simulink model "MX_Gateway"  (Simulink 9.0)
settings: Solver=FixedStepDiscrete  StartTime=0.0  StopTime=100  FixedStep=0.01  RelTol=1e-3
values from neighbouring files (symbols this model uses):
  ACTIVE = Bobcat.Parameter; CreateDefine( ACTIVE                         ,1                          ,'Define' ,'defines.h' )    [defines.m]
  ACTIVE_MODE_ONE = Bobcat.Parameter; CreateDefine( ACTIVE_MODE_ONE                ,19                         ,'Define' ,'defines.h' )    [defines.m]
  AUTHENTICATED = Bobcat.Parameter; CreateDefine( AUTHENTICATED                  ,1                          ,'Define' ,'defines.h' )    [defines.m]
  AUX_EMEA_CONTROLS = Bobcat.Parameter; CreateDefine( AUX_EMEA_CONTROLS  ,1   ,'Define' ,'defines.h' )    [enums.m]
  AUX_FLOW_HIGH = Bobcat.Parameter; CreateDefine( AUX_FLOW_HIGH           ,4  ,'Define' ,'defines.h' )    [enums.m]
  AUX_FLOW_INACTIVE = Bobcat.Parameter; CreateDefine( AUX_FLOW_INACTIVE       ,0  ,'Define' ,'defines.h' )    [enums.m]
  AUX_FLOW_LOW = Bobcat.Parameter; CreateDefine( AUX_FLOW_LOW            ,1  ,'Define' ,'defines.h' )    [enums.m]
  AUX_FLOW_MEDIUM = Bobcat.Parameter; CreateDefine( AUX_FLOW_MEDIUM         ,2  ,'Define' ,'defines.h' )    [enums.m]
  AUX_FLOW_STANDARD = Bobcat.Parameter; CreateDefine( AUX_FLOW_STANDARD       ,3  ,'Define' ,'defines.h' )    [enums.m]
  AUX_NON_FUNCTIONAL = Bobcat.Parameter; CreateDefine( AUX_NON_FUNCTIONAL ,255 ,'Define' ,'defines.h' )    [enums.m]
  BASE_ACTIVE = Bobcat.Parameter; CreateDefine( BASE_ACTIVE                    ,253                        ,'Define' ,'defines.h' )    [defines.m]
  BASE_DETENT = Bobcat.Parameter; CreateDefine( BASE_DETENT                    ,1                          ,'Define' ,'defines.h' )    [defines.m]
  BEEP_NONE = Bobcat.Parameter; CreateDefine( BEEP_NONE                      ,255                        ,'Define' ,'defines.h' )    [defines.m]
  CD_AIR_FILTER_DEBOUNCE_NORMAL = Bobcat.Parameter; CreateAliasCD( CD_AIR_FILTER_DEBOUNCE_NORMAL                        ,uint16(500)                ,'ConstantDataPointer' ,'CONSTANT_DATA' ,'AIR_FILTER_DEBOUNCE_NORMAL')    [cdata.m]    (also in hc12_cdata.m; m4_cdata.m)
  CD_AIR_FILTER_DEBOUNCE_NOT_CONNECTED = Bobcat.Parameter; CreateAliasCD( CD_AIR_FILTER_DEBOUNCE_NOT_CONNECTED                 ,uint16(3000)               ,'ConstantDataPointer' ,'CONSTANT_DATA' ,'AIR_FILTER_DEBOUNCE_NOT_CONNECTED')    [cdata.m]    (also in hc12_cdata.m; m4_cdata.m)
  CD_AIR_FILTER_DEBOUNCE_PLUGGED = Bobcat.Parameter; CreateAliasCD( CD_AIR_FILTER_DEBOUNCE_PLUGGED                       ,uint16(3000)               ,'ConstantDataPointer' ,'CONSTANT_DATA' ,'AIR_FILTER_DEBOUNCE_PLUGGED')    [cdata.m]    (also in hc12_cdata.m; m4_cdata.m)
  CD_AIR_FILTER_FUNCTION = Bobcat.Parameter; CreateAliasCD( CD_AIR_FILTER_FUNCTION                               ,uint8(255)                 ,'ConstantDataPointer' ,'CONSTANT_DATA' ,'AIR_FILTER_FUNCTION')    [cdata.m]    (also in hc12_cdata.m; m4_cdata.m)
  CD_AIR_FILTER_PLUGGED_WEIGHT = Bobcat.Parameter; CreateAliasCD( CD_AIR_FILTER_PLUGGED_WEIGHT                         ,uint8(2)                   ,'ConstantDataPointer' ,'CONSTANT_DATA' ,'AIR_FILTER_PLUGGED_WEIGHT')    [cdata.m]    (also in hc12_cdata.m; m4_cdata.m)
  CD_AIR_FILTER_UNPLUGGED_WEIGHT = Bobcat.Parameter; CreateAliasCD( CD_AIR_FILTER_UNPLUGGED_WEIGHT                       ,uint8(1)                   ,'ConstantDataPointer' ,'CONSTANT_DATA' ,'AIR_FILTER_UNPLUGGED_WEIGHT')    [cdata.m]    (also in hc12_cdata.m; m4_cdata.m)
  CD_AUTOIDLE_ARMBAR_DELAY = Bobcat.Parameter; CreateAliasCD( CD_AUTOIDLE_ARMBAR_DELAY                             ,uint8(2)                   ,'ConstantDataPointer' ,'CONSTANT_DATA' ,'AUTOIDLE_ARMBAR_DELAY')    [cdata.m]    (also in hc12_cdata.m; m4_cdata.m)
  CD_AUTOIDLE_FUNCTION = Bobcat.Parameter; CreateAliasCD( CD_AUTOIDLE_FUNCTION                                 ,uint8(255)                 ,'ConstantDataPointer' ,'CONSTANT_DATA' ,'AUTOIDLE_FUNCTION')    [cdata.m]    (also in hc12_cdata.m; m4_cdata.m)
  CD_AUTOIDLE_IDLE_DELAY = Bobcat.Parameter; CreateAliasCD( CD_AUTOIDLE_IDLE_DELAY                               ,uint8(40)                  ,'ConstantDataPointer' ,'CONSTANT_DATA' ,'AUTOIDLE_IDLE_DELAY')    [cdata.m]    (also in hc12_cdata.m; m4_cdata.m)
  CD_AUTOIDLE_LOW_IDLE_START = Bobcat.Parameter; CreateAliasCD( CD_AUTOIDLE_LOW_IDLE_START                           ,uint8(255)                 ,'ConstantDataPointer' ,'CONSTANT_DATA' ,'AUTOIDLE_LOW_IDLE_START')    [cdata.m]    (also in hc12_cdata.m; m4_cdata.m)
  CD_AUTOIDLE_PRESSURE_OFF = Bobcat.Parameter; CreateAliasCD( CD_AUTOIDLE_PRESSURE_OFF                             ,uint16(300)                ,'ConstantDataPointer' ,'CONSTANT_DATA' ,'AUTOIDLE_PRESSURE_OFF')    [cdata.m]    (also in hc12_cdata.m; m4_cdata.m)
  CD_AUTOIDLE_PRESSURE_ON = Bobcat.Parameter; CreateAliasCD( CD_AUTOIDLE_PRESSURE_ON                              ,uint16(270)                ,'ConstantDataPointer' ,'CONSTANT_DATA' ,'AUTOIDLE_PRESSURE_ON')    [cdata.m]    (also in hc12_cdata.m; m4_cdata.m)
  CD_AUTOSHIFT_FUNCTION = Bobcat.Parameter; CreateAliasCD( CD_AUTOSHIFT_FUNCTION                                ,uint8(255)                 ,'ConstantDataPointer' ,'CONSTANT_DATA' ,'AUTOSHIFT_FUNCTION')    [cdata.m]    (also in hc12_cdata.m; m4_cdata.m)
  CD_AUTOSHIFT_PHIGH1 = Bobcat.Parameter; CreateAliasCD( CD_AUTOSHIFT_PHIGH1                                  ,uint16(205)                ,'ConstantDataPointer' ,'CONSTANT_DATA' ,'AUTOSHIFT_PHIGH1')    [cdata.m]    (also in hc12_cdata.m; m4_cdata.m)
  CD_AUTOSHIFT_PHIGH2 = Bobcat.Parameter; CreateAliasCD( CD_AUTOSHIFT_PHIGH2                                  ,uint16(193)                ,'ConstantDataPointer' ,'CONSTANT_DATA' ,'AUTOSHIFT_PHIGH2')    [cdata.m]    (also in hc12_cdata.m; m4_cdata.m)
  CD_AUTOSHIFT_PHIGH_TEMP1 = Bobcat.Parameter; CreateAliasCD( CD_AUTOSHIFT_PHIGH_TEMP1                             ,int16(70)                  ,'ConstantDataPointer' ,'CONSTANT_DATA' ,'AUTOSHIFT_PHIGH_TEMP1')    [cdata.m]    (also in hc12_cdata.m; m4_cdata.m)
  CD_AUTOSHIFT_PHIGH_TEMP2 = Bobcat.Parameter; CreateAliasCD( CD_AUTOSHIFT_PHIGH_TEMP2                             ,int16(160)                 ,'ConstantDataPointer' ,'CONSTANT_DATA' ,'AUTOSHIFT_PHIGH_TEMP2')    [cdata.m]    (also in hc12_cdata.m; m4_cdata.m)
  CD_AUTOSHIFT_PLOW1 = Bobcat.Parameter; CreateAliasCD( CD_AUTOSHIFT_PLOW1                                   ,uint16(130)                ,'ConstantDataPointer' ,'CONSTANT_DATA' ,'AUTOSHIFT_PLOW1')    [cdata.m]    (also in hc12_cdata.m; m4_cdata.m)
  CD_AUTOSHIFT_PLOW2 = Bobcat.Parameter; CreateAliasCD( CD_AUTOSHIFT_PLOW2                                   ,uint16(100)                ,'ConstantDataPointer' ,'CONSTANT_DATA' ,'AUTOSHIFT_PLOW2')    [cdata.m]    (also in hc12_cdata.m; m4_cdata.m)
  CD_AUTOSHIFT_PLOW_TEMP1 = Bobcat.Parameter; CreateAliasCD( CD_AUTOSHIFT_PLOW_TEMP1                              ,int16(20)                  ,'ConstantDataPointer' ,'CONSTANT_DATA' ,'AUTOSHIFT_PLOW_TEMP1')    [cdata.m]    (also in hc12_cdata.m; m4_cdata.m)
  CD_AUTOSHIFT_PLOW_TEMP2 = Bobcat.Parameter; CreateAliasCD( CD_AUTOSHIFT_PLOW_TEMP2                              ,int16(80)                  ,'ConstantDataPointer' ,'CONSTANT_DATA' ,'AUTOSHIFT_PLOW_TEMP2')    [cdata.m]    (also in hc12_cdata.m; m4_cdata.m)
  CD_AUTOSHIFT_TRAVEL_DELAY = Bobcat.Parameter; CreateAliasCD( CD_AUTOSHIFT_TRAVEL_DELAY                            ,uint8(32)                  ,'ConstantDataPointer' ,'CONSTANT_DATA' ,'AUTOSHIFT_TRAVEL_DELAY')    [cdata.m]    (also in hc12_cdata.m; m4_cdata.m)
  CD_AUX2_CRACKPOINT_CURRENT = Bobcat.Parameter; CreateAliasCD( CD_AUX2_CRACKPOINT_CURRENT                           ,fi(0,0,16,12,'hex','0c28') ,'ConstantDataPointer' ,'CONSTANT_DATA' ,'AUX2_CRACKPOINT_CURRENT')    [cdata.m]    (also in hc12_cdata.m; m4_cdata.m)
  CD_AUX2_FLOW_LOW_FUNCTION = Bobcat.Parameter; CreateAliasCD( CD_AUX2_FLOW_LOW_FUNCTION                            ,uint8(255)                 ,'ConstantDataPointer' ,'CONSTANT_DATA' ,'AUX2_FLOW_LOW_FUNCTION')    [cdata.m]    (also in hc12_cdata.m; m4_cdata.m)
  CD_AUX2_FLOW_MED_FUNCTION = Bobcat.Parameter; CreateAliasCD( CD_AUX2_FLOW_MED_FUNCTION                            ,uint8(255)                 ,'ConstantDataPointer' ,'CONSTANT_DATA' ,'AUX2_FLOW_MED_FUNCTION')    [cdata.m]    (also in hc12_cdata.m; m4_cdata.m)
  CD_AUX2_HI_FLOW_PERCENT = Bobcat.Parameter; CreateAliasCD( CD_AUX2_HI_FLOW_PERCENT                              ,fi(0,0,16,16,'hex','ffff') ,'ConstantDataPointer' ,'CONSTANT_DATA' ,'AUX2_HI_FLOW_PERCENT')    [cdata.m]    (also in hc12_cdata.m; m4_cdata.m)
  CD_AUX2_LOW_FLOW_PERCENT = Bobcat.Parameter; CreateAliasCD( CD_AUX2_LOW_FLOW_PERCENT                             ,fi(0,0,16,16,'hex','7333') ,'ConstantDataPointer' ,'CONSTANT_DATA' ,'AUX2_LOW_FLOW_PERCENT')    [cdata.m]    (also in hc12_cdata.m; m4_cdata.m)
  CD_AUX2_MAX_FLOW_CURRENT = Bobcat.Parameter; CreateAliasCD( CD_AUX2_MAX_FLOW_CURRENT                             ,fi(0,0,16,12,'hex','14cc') ,'ConstantDataPointer' ,'CONSTANT_DATA' ,'AUX2_MAX_FLOW_CURRENT')    [cdata.m]    (also in hc12_cdata.m; m4_cdata.m)
  CD_AUX2_MED_FLOW_PERCENT = Bobcat.Parameter; CreateAliasCD( CD_AUX2_MED_FLOW_PERCENT                             ,fi(0,0,16,16,'hex','9999') ,'ConstantDataPointer' ,'CONSTANT_DATA' ,'AUX2_MED_FLOW_PERCENT')    [cdata.m]    (also in hc12_cdata.m; m4_cdata.m)
  CD_AUX_CRACKPOINT_CURRENT = Bobcat.Parameter; CreateAliasCD( CD_AUX_CRACKPOINT_CURRENT                            ,fi(0,0,16,12,'hex','0000') ,'ConstantDataPointer' ,'CONSTANT_DATA' ,'AUX_CRACKPOINT_CURRENT')    [cdata.m]    (also in hc12_cdata.m; m4_cdata.m)
  CD_AUX_DETENT_FLASH_TIME = Bobcat.Parameter; CreateAliasCD( CD_AUX_DETENT_FLASH_TIME                             ,uint16(72)                 ,'ConstantDataPointer' ,'CONSTANT_DATA' ,'AUX_DETENT_FLASH_TIME')    [cdata.m]    (also in hc12_cdata.m; m4_cdata.m)
  CD_AUX_DETENT_HOLD_TIME = Bobcat.Parameter; CreateAliasCD( CD_AUX_DETENT_HOLD_TIME                              ,uint16(100)                ,'ConstantDataPointer' ,'CONSTANT_DATA' ,'AUX_DETENT_HOLD_TIME')    [cdata.m]    (also in hc12_cdata.m; m4_cdata.m)
  CD_AUX_FULL_ON_AUX1 = Bobcat.Parameter; CreateAliasCD( CD_AUX_FULL_ON_AUX1                                  ,fi(0,0,16,12,'hex','10cd') ,'ConstantDataPointer' ,'CONSTANT_DATA' ,'AUX_FULL_ON_AUX1')    [cdata.m]    (also in hc12_cdata.m; m4_cdata.m)
  CD_AUX_FULL_ON_AUX2 = Bobcat.Parameter; CreateAliasCD( CD_AUX_FULL_ON_AUX2                                  ,fi(0,0,16,12,'hex','139d') ,'ConstantDataPointer' ,'CONSTANT_DATA' ,'AUX_FULL_ON_AUX2')    [cdata.m]    (also in hc12_cdata.m; m4_cdata.m)
  CD_AUX_FULL_ON_AUX3 = Bobcat.Parameter; CreateAliasCD( CD_AUX_FULL_ON_AUX3                                  ,fi(0,0,16,12,'hex','17d7') ,'ConstantDataPointer' ,'CONSTANT_DATA' ,'AUX_FULL_ON_AUX3')    [cdata.m]    (also in hc12_cdata.m; m4_cdata.m)
  CD_AUX_FUNCTION = Bobcat.Parameter; CreateAliasCD( CD_AUX_FUNCTION                                      ,uint8(0)                   ,'ConstantDataPointer' ,'CONSTANT_DATA' ,'AUX_FUNCTION')    [cdata.m]    (also in hc12_cdata.m; m4_cdata.m)
  CD_AUX_RATE_LIMIT_CRACKPOINT = Bobcat.Parameter; CreateAliasCD( CD_AUX_RATE_LIMIT_CRACKPOINT                         ,fi(0,0,16,12,'hex','0800') ,'ConstantDataPointer' ,'CONSTANT_DATA' ,'AUX_RATE_LIMIT_CRACKPOINT')    [cdata.m]    (also in hc12_cdata.m; m4_cdata.m)
  CD_AUX_RATE_LIMIT_CRACKPOINT_RATE = Bobcat.Parameter; CreateAliasCD( CD_AUX_RATE_LIMIT_CRACKPOINT_RATE                    ,fi(0,1,32,12,'int',102400) ,'ConstantDataPointer' ,'CONSTANT_DATA' ,'AUX_RATE_LIMIT_CRACKPOINT_RATE')    [cdata.m]    (also in hc12_cdata.m; m4_cdata.m)
  CD_AUX_RATE_LIMIT_FUNCTION = Bobcat.Parameter; CreateAliasCD( CD_AUX_RATE_LIMIT_FUNCTION                           ,uint8(255)                 ,'ConstantDataPointer' ,'CONSTANT_DATA' ,'AUX_RATE_LIMIT_FUNCTION')    [cdata.m]    (also in hc12_cdata.m; m4_cdata.m)
  CD_AUX_RATE_LIMIT_MAX_ENG_SPD = Bobcat.Parameter; CreateAliasCD( CD_AUX_RATE_LIMIT_MAX_ENG_SPD                        ,uint16(1600)               ,'ConstantDataPointer' ,'CONSTANT_DATA' ,'AUX_RATE_LIMIT_MAX_ENG_SPD')    [cdata.m]    (also in hc12_cdata.m; m4_cdata.m)
  CD_AUX_RATE_LIMIT_MIN_ENG_SPD = Bobcat.Parameter; CreateAliasCD( CD_AUX_RATE_LIMIT_MIN_ENG_SPD                        ,uint16(100)                ,'ConstantDataPointer' ,'CONSTANT_DATA' ,'AUX_RATE_LIMIT_MIN_ENG_SPD')    [cdata.m]    (also in hc12_cdata.m; m4_cdata.m)
  CD_AUX_RATE_LIMIT_NORMAL_RATE = Bobcat.Parameter; CreateAliasCD( CD_AUX_RATE_LIMIT_NORMAL_RATE                        ,fi(0,1,32,12,'int',2048)   ,'ConstantDataPointer' ,'CONSTANT_DATA' ,'AUX_RATE_LIMIT_NORMAL_RATE')    [cdata.m]    (also in hc12_cdata.m; m4_cdata.m)
  CD_AUXPOT_CAL_DEADBAND_HIGH = Bobcat.Parameter; CreateAliasCD( CD_AUXPOT_CAL_DEADBAND_HIGH                          ,uint16(574)                ,'ConstantDataPointer' ,'CONSTANT_DATA' ,'AUXPOT_CAL_DEADBAND_HIGH')    [cdata.m]    (also in hc12_cdata.m; m4_cdata.m)
  CD_AUXPOT_CAL_DEADBAND_LOW = Bobcat.Parameter; CreateAliasCD( CD_AUXPOT_CAL_DEADBAND_LOW                           ,uint16(424)                ,'ConstantDataPointer' ,'CONSTANT_DATA' ,'AUXPOT_CAL_DEADBAND_LOW')    [cdata.m]    (also in hc12_cdata.m; m4_cdata.m)
  CD_AUXPOT_CENTER_RATIO = Bobcat.Parameter; CreateAliasCD( CD_AUXPOT_CENTER_RATIO                               ,uint16(500)                ,'ConstantDataPointer' ,'CONSTANT_DATA' ,'AUXPOT_CENTER_RATIO')    [cdata.m]    (also in hc12_cdata.m; m4_cdata.m)
  CD_AUXPOT_DEADBAND = Bobcat.Parameter; CreateAliasCD( CD_AUXPOT_DEADBAND                                   ,uint8(38)                  ,'ConstantDataPointer' ,'CONSTANT_DATA' ,'AUXPOT_DEADBAND')    [cdata.m]    (also in hc12_cdata.m; m4_cdata.m)
  CD_AUXPOT_FUNCTION = Bobcat.Parameter; CreateAliasCD( CD_AUXPOT_FUNCTION                                   ,uint8(0)                   ,'ConstantDataPointer' ,'CONSTANT_DATA' ,'AUXPOT_FUNCTION')    [cdata.m]    (also in hc12_cdata.m; m4_cdata.m)
  CD_AUXPOT_RATIO_GAIN = Bobcat.Parameter; CreateAliasCD( CD_AUXPOT_RATIO_GAIN                                 ,uint16(1000)               ,'ConstantDataPointer' ,'CONSTANT_DATA' ,'AUXPOT_RATIO_GAIN')    [cdata.m]    (also in hc12_cdata.m; m4_cdata.m)
  CD_CAN_THROTTLE_FUNCTION = Bobcat.Parameter; CreateAliasCD( CD_CAN_THROTTLE_FUNCTION                             ,uint8(255)                 ,'ConstantDataPointer' ,'CONSTANT_DATA' ,'CAN_THROTTLE_FUNCTION')    [cdata.m]    (also in hc12_cdata.m; m4_cdata.m)
  CD_CAN_THROTTLE_REVERSE = Bobcat.Parameter; CreateAliasCD( CD_CAN_THROTTLE_REVERSE                              ,uint8(255)                 ,'ConstantDataPointer' ,'CONSTANT_DATA' ,'CAN_THROTTLE_REVERSE')    [cdata.m]    (also in hc12_cdata.m; m4_cdata.m)
  CD_CONSOLE_DEBOUNCE_ABSENT = Bobcat.Parameter; CreateAliasCD( CD_CONSOLE_DEBOUNCE_ABSENT                           ,uint16(12)                 ,'ConstantDataPointer' ,'CONSTANT_DATA' ,'CONSOLE_DEBOUNCE_ABSENT')    [cdata.m]    (also in hc12_cdata.m; m4_cdata.m)
  CD_CONSOLE_DEBOUNCE_OORH = Bobcat.Parameter; CreateAliasCD( CD_CONSOLE_DEBOUNCE_OORH                             ,uint16(12)                 ,'ConstantDataPointer' ,'CONSTANT_DATA' ,'CONSOLE_DEBOUNCE_OORH')    [cdata.m]    (also in hc12_cdata.m; m4_cdata.m)
  CD_CONSOLE_DEBOUNCE_OORL = Bobcat.Parameter; CreateAliasCD( CD_CONSOLE_DEBOUNCE_OORL                             ,uint16(12)                 ,'ConstantDataPointer' ,'CONSTANT_DATA' ,'CONSOLE_DEBOUNCE_OORL')    [cdata.m]    (also in hc12_cdata.m; m4_cdata.m)
  CD_CONSOLE_DEBOUNCE_PRESENT = Bobcat.Parameter; CreateAliasCD( CD_CONSOLE_DEBOUNCE_PRESENT                          ,uint16(12)                 ,'ConstantDataPointer' ,'CONSTANT_DATA' ,'CONSOLE_DEBOUNCE_PRESENT')    [cdata.m]    (also in hc12_cdata.m; m4_cdata.m)
  CD_CONSOLE_FUNCTION = Bobcat.Parameter; CreateAliasCD( CD_CONSOLE_FUNCTION                                  ,uint8(0)                   ,'ConstantDataPointer' ,'CONSTANT_DATA' ,'CONSOLE_FUNCTION')    [cdata.m]    (also in hc12_cdata.m; m4_cdata.m)
  CD_CONSOLE_OORH = Bobcat.Parameter; CreateAliasCD( CD_CONSOLE_OORH                                      ,uint16(740)                ,'ConstantDataPointer' ,'CONSTANT_DATA' ,'CONSOLE_OORH')    [cdata.m]    (also in hc12_cdata.m; m4_cdata.m)
  CD_CONSOLE_OORL = Bobcat.Parameter; CreateAliasCD( CD_CONSOLE_OORL                                      ,uint16(10)                 ,'ConstantDataPointer' ,'CONSTANT_DATA' ,'CONSOLE_OORL')    [cdata.m]    (also in hc12_cdata.m; m4_cdata.m)
  CD_CONSOLE_PRESENT_HIGH = Bobcat.Parameter; CreateAliasCD( CD_CONSOLE_PRESENT_HIGH                              ,uint16(515)                ,'ConstantDataPointer' ,'CONSTANT_DATA' ,'CONSOLE_PRESENT_HIGH')    [cdata.m]    (also in hc12_cdata.m; m4_cdata.m)
  CD_CONSOLE_PRESENT_LOW = Bobcat.Parameter; CreateAliasCD( CD_CONSOLE_PRESENT_LOW                               ,uint16(375)                ,'ConstantDataPointer' ,'CONSTANT_DATA' ,'CONSOLE_PRESENT_LOW')    [cdata.m]    (also in hc12_cdata.m; m4_cdata.m)
  CD_DELUXE_GEN5_5_FUNCTION = Bobcat.Parameter; CreateAliasCD( CD_DELUXE_GEN5_5_FUNCTION                            ,uint8(255)                 ,'ConstantDataPointer' ,'CONSTANT_DATA' ,'DELUXE_GEN5_5_FUNCTION')    [cdata.m]    (also in hc12_cdata.m; m4_cdata.m)
  CD_DELUXE_GEN5_FUNCTION = Bobcat.Parameter; CreateAliasCD( CD_DELUXE_GEN5_FUNCTION                              ,uint8(255)                 ,'ConstantDataPointer' ,'CONSTANT_DATA' ,'DELUXE_GEN5_FUNCTION')    [cdata.m]    (also in hc12_cdata.m; m4_cdata.m)
  CD_DERATE_ENGINE_SPEED_DEBOUNCE_HIGH = Bobcat.Parameter; CreateAliasCD( CD_DERATE_ENGINE_SPEED_DEBOUNCE_HIGH                 ,uint16(200)                ,'ConstantDataPointer' ,'CONSTANT_DATA' ,'DERATE_ENGINE_SPEED_DEBOUNCE_HIGH')    [cdata.m]    (also in hc12_cdata.m; m4_cdata.m)
  CD_DERATE_ENGINE_SPEED_DEBOUNCE_SHUTDOWN = Bobcat.Parameter; CreateAliasCD( CD_DERATE_ENGINE_SPEED_DEBOUNCE_SHUTDOWN             ,uint16(1000)               ,'ConstantDataPointer' ,'CONSTANT_DATA' ,'DERATE_ENGINE_SPEED_DEBOUNCE_SHUTDOWN')    [cdata.m]    (also in hc12_cdata.m; m4_cdata.m)
  CD_DERATE_ENGINE_SPEED_HIGH_OFFSET = Bobcat.Parameter; CreateAliasCD( CD_DERATE_ENGINE_SPEED_HIGH_OFFSET                   ,uint16(200)                ,'ConstantDataPointer' ,'CONSTANT_DATA' ,'DERATE_ENGINE_SPEED_HIGH_OFFSET')    [cdata.m]    (also in hc12_cdata.m; m4_cdata.m)
  CD_DERATE_METHOD = Bobcat.Parameter; CreateAliasCD( CD_DERATE_METHOD                                     ,uint8(0)                   ,'ConstantDataPointer' ,'CONSTANT_DATA' ,'DERATE_METHOD')    [cdata.m]    (also in hc12_cdata.m; m4_cdata.m)
  CD_DUMP_VALVE_FUNCTION = Bobcat.Parameter; CreateAliasCD( CD_DUMP_VALVE_FUNCTION                               ,uint8(255)                 ,'ConstantDataPointer' ,'CONSTANT_DATA' ,'DUMP_VALVE_FUNCTION')    [cdata.m]    (also in hc12_cdata.m; m4_cdata.m)
  CD_DUMP_VALVE_ON_CURRENT = Bobcat.Parameter; CreateAliasCD( CD_DUMP_VALVE_ON_CURRENT                             ,fi(0,0,16,12,'hex','ffff') ,'ConstantDataPointer' ,'CONSTANT_DATA' ,'DUMP_VALVE_ON_CURRENT')    [cdata.m]    (also in hc12_cdata.m; m4_cdata.m)
  CD_ECO_MODE_THROTTLE_REDUCTION = Bobcat.Parameter; CreateAliasCD( CD_ECO_MODE_THROTTLE_REDUCTION                       ,uint16(850)                ,'ConstantDataPointer' ,'CONSTANT_DATA' ,'ECO_MODE_THROTTLE_REDUCTION')    [cdata.m]    (also in hc12_cdata.m; m4_cdata.m)
  CD_ECT_FILTER_GAIN = Bobcat.Parameter; CreateAliasCD( CD_ECT_FILTER_GAIN                                   ,uint8(6)                   ,'ConstantDataPointer' ,'CONSTANT_DATA' ,'ECT_FILTER_GAIN')    [cdata.m]    (also in hc12_cdata.m; m4_cdata.m)
  CD_ECU_FUNCTION = Bobcat.Parameter; CreateAliasCD( CD_ECU_FUNCTION                                      ,uint8(255)                 ,'ConstantDataPointer' ,'CONSTANT_DATA' ,'ECU_FUNCTION')    [cdata.m]    (also in hc12_cdata.m; m4_cdata.m)
  CD_ECU_HOLD_DEBOUNCE_ERROR_ON = Bobcat.Parameter; CreateAliasCD( CD_ECU_HOLD_DEBOUNCE_ERROR_ON                        ,uint16(20)                 ,'ConstantDataPointer' ,'CONSTANT_DATA' ,'ECU_HOLD_DEBOUNCE_ERROR_ON')    [cdata.m]    (also in hc12_cdata.m; m4_cdata.m)
  CD_EMEA_CONTROLS_HOLD_TIME = Bobcat.Parameter; CreateAliasCD( CD_EMEA_CONTROLS_HOLD_TIME                           ,uint16(30)                 ,'ConstantDataPointer' ,'CONSTANT_DATA' ,'EMEA_CONTROLS_HOLD_TIME')    [cdata.m]    (also in hc12_cdata.m; m4_cdata.m)
  CD_EMEA_CONTROLS_OPTION_A = Bobcat.Parameter; CreateAliasCD( CD_EMEA_CONTROLS_OPTION_A                            ,uint8(255)                 ,'ConstantDataPointer' ,'CONSTANT_DATA' ,'EMEA_CONTROLS_OPTION_A')    [cdata.m]    (also in hc12_cdata.m; m4_cdata.m)
  CD_EMEA_CONTROLS_OPTION_C = Bobcat.Parameter; CreateAliasCD( CD_EMEA_CONTROLS_OPTION_C                            ,uint8(255)                 ,'ConstantDataPointer' ,'CONSTANT_DATA' ,'EMEA_CONTROLS_OPTION_C')    [cdata.m]    (also in hc12_cdata.m; m4_cdata.m)
  CD_EMEA_CONTROLS_REVERSE = Bobcat.Parameter; CreateAliasCD( CD_EMEA_CONTROLS_REVERSE                             ,uint8(255)                 ,'ConstantDataPointer' ,'CONSTANT_DATA' ,'EMEA_CONTROLS_REVERSE')    [cdata.m]    (also in hc12_cdata.m; m4_cdata.m)
  CD_EMEA_ELECTRIC_VERSION = Bobcat.Parameter; CreateAliasCD( CD_EMEA_ELECTRIC_VERSION                             ,uint8(0)                   ,'ConstantDataPointer' ,'CONSTANT_DATA' ,'EMEA_ELECTRIC_VERSION')    [cdata.m]    (also in hc12_cdata.m; m4_cdata.m)
  CD_EMEA_POT_CAL_DEADBAND_HIGH = Bobcat.Parameter; CreateAliasCD( CD_EMEA_POT_CAL_DEADBAND_HIGH                        ,uint16(574)                ,'ConstantDataPointer' ,'CONSTANT_DATA' ,'EMEA_POT_CAL_DEADBAND_HIGH')    [cdata.m]    (also in hc12_cdata.m; m4_cdata.m)
  CD_EMEA_POT_CAL_DEADBAND_LOW = Bobcat.Parameter; CreateAliasCD( CD_EMEA_POT_CAL_DEADBAND_LOW                         ,uint16(424)                ,'ConstantDataPointer' ,'CONSTANT_DATA' ,'EMEA_POT_CAL_DEADBAND_LOW')    [cdata.m]    (also in hc12_cdata.m; m4_cdata.m)
  CD_ENGINE_RPM_FACTOR = Bobcat.Parameter; CreateAliasCD( CD_ENGINE_RPM_FACTOR                                 ,uint8(35)                  ,'ConstantDataPointer' ,'CONSTANT_DATA' ,'ENGINE_RPM_FACTOR')    [cdata.m]    (also in hc12_cdata.m; m4_cdata.m)
  CD_ENGINE_SPEED_FILTER_FAST_VALUE = Bobcat.Parameter; CreateAliasCD( CD_ENGINE_SPEED_FILTER_FAST_VALUE                    ,uint8(1)                   ,'ConstantDataPointer' ,'CONSTANT_DATA' ,'ENGINE_SPEED_FILTER_FAST_VALUE')    [cdata.m]    (also in hc12_cdata.m; m4_cdata.m)
  CD_ENGINE_SPEED_FILTER_SLOW_VALUE = Bobcat.Parameter; CreateAliasCD( CD_ENGINE_SPEED_FILTER_SLOW_VALUE                    ,uint8(4)                   ,'ConstantDataPointer' ,'CONSTANT_DATA' ,'ENGINE_SPEED_FILTER_SLOW_VALUE')    [cdata.m]    (also in hc12_cdata.m; m4_cdata.m)
  CD_ENGINE_SPEED_RUNNING = Bobcat.Parameter; CreateAliasCD( CD_ENGINE_SPEED_RUNNING                              ,uint16(800)                ,'ConstantDataPointer' ,'CONSTANT_DATA' ,'ENGINE_SPEED_RUNNING')    [cdata.m]    (also in hc12_cdata.m; m4_cdata.m)
  CD_ENGINE_START_DELAY_DEBOUNCE_RPM_NO_SIGNAL = Bobcat.Parameter; CreateAliasCD( CD_ENGINE_START_DELAY_DEBOUNCE_RPM_NO_SIGNAL         ,uint16(0)                  ,'ConstantDataPointer' ,'CONSTANT_DATA' ,'ENGINE_START_DELAY_DEBOUNCE_RPM_NO_SIGNAL')    [cdata.m]    (also in hc12_cdata.m; m4_cdata.m)
  CD_ENGINE_START_DELAY_OFF_TIME = Bobcat.Parameter; CreateAliasCD( CD_ENGINE_START_DELAY_OFF_TIME                       ,uint16(0)                  ,'ConstantDataPointer' ,'CONSTANT_DATA' ,'ENGINE_START_DELAY_OFF_TIME')    [cdata.m]    (also in hc12_cdata.m; m4_cdata.m)
  CD_ENGINE_START_DELAY_RPM_NO_SIGNAL = Bobcat.Parameter; CreateAliasCD( CD_ENGINE_START_DELAY_RPM_NO_SIGNAL                  ,uint16(0)                  ,'ConstantDataPointer' ,'CONSTANT_DATA' ,'ENGINE_START_DELAY_RPM_NO_SIGNAL')    [cdata.m]    (also in hc12_cdata.m; m4_cdata.m)
  CD_EOP_DEBOUNCE_EXTREMELY_LOW = Bobcat.Parameter; CreateAliasCD( CD_EOP_DEBOUNCE_EXTREMELY_LOW                        ,uint16(50)                 ,'ConstantDataPointer' ,'CONSTANT_DATA' ,'EOP_DEBOUNCE_EXTREMELY_LOW')    [cdata.m]    (also in hc12_cdata.m; m4_cdata.m)
  CD_EOP_DEBOUNCE_NORMAL = Bobcat.Parameter; CreateAliasCD( CD_EOP_DEBOUNCE_NORMAL                               ,uint16(50)                 ,'ConstantDataPointer' ,'CONSTANT_DATA' ,'EOP_DEBOUNCE_NORMAL')    [cdata.m]    (also in hc12_cdata.m; m4_cdata.m)
  CD_EOP_DEBOUNCE_SHUTDOWN = Bobcat.Parameter; CreateAliasCD( CD_EOP_DEBOUNCE_SHUTDOWN                             ,uint16(100)                ,'ConstantDataPointer' ,'CONSTANT_DATA' ,'EOP_DEBOUNCE_SHUTDOWN')    [cdata.m]    (also in hc12_cdata.m; m4_cdata.m)
  CD_EOP_FILTER_VALUE = Bobcat.Parameter; CreateAliasCD( CD_EOP_FILTER_VALUE                                  ,uint8(3)                   ,'ConstantDataPointer' ,'CONSTANT_DATA' ,'EOP_FILTER_VALUE')    [cdata.m]    (also in hc12_cdata.m; m4_cdata.m)
  CD_EOP_FUNCTION = Bobcat.Parameter; CreateAliasCD( CD_EOP_FUNCTION                                      ,uint8(1)                   ,'ConstantDataPointer' ,'CONSTANT_DATA' ,'EOP_FUNCTION')    [cdata.m]    (also in hc12_cdata.m; m4_cdata.m)
  CD_EOP_SWITCH_GAUGE_VALUE = Bobcat.Parameter; CreateAliasCD( CD_EOP_SWITCH_GAUGE_VALUE                            ,uint16(125)                ,'ConstantDataPointer' ,'CONSTANT_DATA' ,'EOP_SWITCH_GAUGE_VALUE')    [cdata.m]    (also in hc12_cdata.m; m4_cdata.m)
  CD_EOP_SWITCH_LOW = Bobcat.Parameter; CreateAliasCD( CD_EOP_SWITCH_LOW                                    ,uint16(50)                 ,'ConstantDataPointer' ,'CONSTANT_DATA' ,'EOP_SWITCH_LOW')    [cdata.m]    (also in hc12_cdata.m; m4_cdata.m)
  CD_EOP_SWITCH_PRESSURE_VALUE = Bobcat.Parameter; CreateAliasCD( CD_EOP_SWITCH_PRESSURE_VALUE                         ,uint16(40)                 ,'ConstantDataPointer' ,'CONSTANT_DATA' ,'EOP_SWITCH_PRESSURE_VALUE')    [cdata.m]    (also in hc12_cdata.m; m4_cdata.m)
  CD_EOT_DEBOUNCE_EXTREMELY_LOW = Bobcat.Parameter; CreateAliasCD( CD_EOT_DEBOUNCE_EXTREMELY_LOW                        ,uint16(2)                  ,'ConstantDataPointer' ,'CONSTANT_DATA' ,'EOT_DEBOUNCE_EXTREMELY_LOW')    [cdata.m]    (also in hc12_cdata.m; m4_cdata.m)
  CD_EOT_DEBOUNCE_LOW = Bobcat.Parameter; CreateAliasCD( CD_EOT_DEBOUNCE_LOW                                  ,uint16(2)                  ,'ConstantDataPointer' ,'CONSTANT_DATA' ,'EOT_DEBOUNCE_LOW')    [cdata.m]    (also in hc12_cdata.m; m4_cdata.m)
  CD_EOT_DEBOUNCE_NORMAL = Bobcat.Parameter; CreateAliasCD( CD_EOT_DEBOUNCE_NORMAL                               ,uint16(2)                  ,'ConstantDataPointer' ,'CONSTANT_DATA' ,'EOT_DEBOUNCE_NORMAL')    [cdata.m]    (also in hc12_cdata.m; m4_cdata.m)
  CD_EOT_EXTREMELY_LOW = Bobcat.Parameter; CreateAliasCD( CD_EOT_EXTREMELY_LOW                                 ,uint16(81)                 ,'ConstantDataPointer' ,'CONSTANT_DATA' ,'EOT_EXTREMELY_LOW')    [cdata.m]    (also in hc12_cdata.m; m4_cdata.m)
  CD_EOT_FUNCTION = Bobcat.Parameter; CreateAliasCD( CD_EOT_FUNCTION                                      ,uint8(255)                 ,'ConstantDataPointer' ,'CONSTANT_DATA' ,'EOT_FUNCTION')    [cdata.m]    (also in hc12_cdata.m; m4_cdata.m)
  CD_EOT_LOW = Bobcat.Parameter; CreateAliasCD( CD_EOT_LOW                                           ,uint16(90)                 ,'ConstantDataPointer' ,'CONSTANT_DATA' ,'EOT_LOW')    [cdata.m]    (also in hc12_cdata.m; m4_cdata.m)
  CD_EOT_TEMPERATURE_HYSTERESIS = Bobcat.Parameter; CreateAliasCD( CD_EOT_TEMPERATURE_HYSTERESIS                        ,uint16(2)                  ,'ConstantDataPointer' ,'CONSTANT_DATA' ,'EOT_TEMPERATURE_HYSTERESIS')    [cdata.m]    (also in hc12_cdata.m; m4_cdata.m)
  CD_FAST_TRAVEL_FUNCTION = Bobcat.Parameter; CreateAliasCD( CD_FAST_TRAVEL_FUNCTION                              ,uint8(255)                 ,'ConstantDataPointer' ,'CONSTANT_DATA' ,'FAST_TRAVEL_FUNCTION')    [cdata.m]    (also in hc12_cdata.m; m4_cdata.m)
  CD_FAST_TRAVEL_THROTTLE_DELAY = Bobcat.Parameter; CreateAliasCD( CD_FAST_TRAVEL_THROTTLE_DELAY                        ,uint16(10)                 ,'ConstantDataPointer' ,'CONSTANT_DATA' ,'FAST_TRAVEL_THROTTLE_DELAY')    [cdata.m]    (also in hc12_cdata.m; m4_cdata.m)
  CD_FAST_TRAVEL_THROTTLE_LIMIT = Bobcat.Parameter; CreateAliasCD( CD_FAST_TRAVEL_THROTTLE_LIMIT                        ,uint16(1000)               ,'ConstantDataPointer' ,'CONSTANT_DATA' ,'FAST_TRAVEL_THROTTLE_LIMIT')    [cdata.m]    (also in hc12_cdata.m; m4_cdata.m)
  CD_FLYWHEEL_TEETH = Bobcat.Parameter; CreateAliasCD( CD_FLYWHEEL_TEETH                                    ,uint8(83)                  ,'ConstantDataPointer' ,'CONSTANT_DATA' ,'FLYWHEEL_TEETH')    [cdata.m]    (also in hc12_cdata.m; m4_cdata.m)
  CD_FUEL_LEVEL_FILTER_FAST_VALUE = Bobcat.Parameter; CreateAliasCD( CD_FUEL_LEVEL_FILTER_FAST_VALUE                      ,uint8(5)                   ,'ConstantDataPointer' ,'CONSTANT_DATA' ,'FUEL_LEVEL_FILTER_FAST_VALUE')    [cdata.m]    (also in hc12_cdata.m; m4_cdata.m)
  CD_FUEL_LEVEL_FILTER_SLOW_VALUE = Bobcat.Parameter; CreateAliasCD( CD_FUEL_LEVEL_FILTER_SLOW_VALUE                      ,uint8(10)                  ,'ConstantDataPointer' ,'CONSTANT_DATA' ,'FUEL_LEVEL_FILTER_SLOW_VALUE')    [cdata.m]    (also in hc12_cdata.m; m4_cdata.m)
  CD_FUEL_PRIMARY_FEEDBACK_FUNCTION = Bobcat.Parameter; CreateAliasCD( CD_FUEL_PRIMARY_FEEDBACK_FUNCTION                    ,uint8(0)                   ,'ConstantDataPointer' ,'CONSTANT_DATA' ,'FUEL_PRIMARY_FEEDBACK_FUNCTION')    [cdata.m]    (also in hc12_cdata.m; m4_cdata.m)
  ... (+246 more)
undefined symbols (not defined in the file or in its folder): sigbuilder_block
undefined symbols (not defined in the file): CD_FUEL_RESOLUTION_TIMER
libraries: simulink x186, MX_Library x107
(part 39 of 48)

== stateflow chart MX_Gtwy_Control/Inputs/AirFilter/Filter ==
  state PERSISTANCE_COUNTER [parallel]
    settings: executionOrder=1
    state COUNTER
      en:count_AFPlugged = 0;
      en:state_Engine_old = state_Engine;
      state OFF
        OFF -> (junction 4037) [order 1]
        (junction 4037) -> (junction 4038) [order 1] : [(count_AFPlugged >0)]
        (junction 4037) -> (junction 4039) [order 2]
        (junction 4038) -> (junction 4040) : {
            | count_AFPlugged = count_AFPlugged - CD_AIR_FILTER_UNPLUGGED_WEIGHT;
            | }
        (junction 4040) -> (junction 4039)
      state ON
        ON -> (junction 4046) [order 1]
        (junction 4046) -> (junction 4047) [order 1] : [(count_AFPlugged < 65535)]
        (junction 4046) -> (junction 4048) [order 2]
        (junction 4047) -> (junction 4049) : {
            | count_AFPlugged = count_AFPlugged + CD_AIR_FILTER_PLUGGED_WEIGHT;
            | }
        (junction 4049) -> (junction 4048)
      (default) -> OFF
      OFF -> ON [order 1] : [(din_AirFilter > 0)]
      ON -> OFF [order 1] : [(din_AirFilter == 0)]
    (default) -> COUNTER
    COUNTER -> COUNTER : [(state_Engine_old != state_Engine)]
  state FAULT_DETECTION [parallel]
    settings: executionOrder=2
    state NON_FUNCTIONAL
      en:state_AirFilter = NORMAL;
    state COUNTER_BASED
      state NOT_CONNECTED
        en:state_AirFilter = NOT_CONNECTED;
      state NORMAL
        en:state_AirFilter = NORMAL;
      state PLUGGED
        en:state_AirFilter = PLUGGED;
      (default) -> FAULT_DETECTION.COUNTER_BASED.NORMAL
      FAULT_DETECTION.COUNTER_BASED.NORMAL -> FAULT_DETECTION.COUNTER_BASED.NOT_CONNECTED [order 2] : [((state_Engine == ENGINE_NOT_RUNNING) || (state_AirFilter == NOT_CONNECTED))...
          | && (count_AFPlugged >= CD_AIR_FILTER_DEBOUNCE_NOT_CONNECTED)]
      FAULT_DETECTION.COUNTER_BASED.NOT_CONNECTED -> FAULT_DETECTION.COUNTER_BASED.NORMAL : [(count_AFPlugged < CD_AIR_FILTER_DEBOUNCE_NOT_CONNECTED)]
      FAULT_DETECTION.COUNTER_BASED.NORMAL -> FAULT_DETECTION.COUNTER_BASED.PLUGGED [order 1] : [(state_Engine == ENGINE_RUNNING)...
          | && (count_AFPlugged >= CD_AIR_FILTER_DEBOUNCE_PLUGGED)]
      FAULT_DETECTION.COUNTER_BASED.PLUGGED -> FAULT_DETECTION.COUNTER_BASED.NORMAL : [(count_AFPlugged < CD_AIR_FILTER_DEBOUNCE_PLUGGED)...
          | && (state_AirFilter == NORMAL)]
    state TIME_BASED
      state NOT_CONNECTED
        state NOT_CONNECTED
          en:state_AirFilter = NOT_CONNECTED;
        state DEBOUNCE
          en:debounceCount = 0;
          du:debounceCount++;
        (default) -> FAULT_DETECTION.TIME_BASED.NOT_CONNECTED.DEBOUNCE
        FAULT_DETECTION.TIME_BASED.NOT_CONNECTED.DEBOUNCE -> FAULT_DETECTION.TIME_BASED.NOT_CONNECTED.NOT_CONNECTED : [(debounceCount >= CD_AIR_FILTER_DEBOUNCE_NOT_CONNECTED)...
            | || (state_AirFilter == NOT_CONNECTED)]
      state NORMAL
        state DEBOUNCE
          en:debounceCount = 0;
          du:debounceCount++;
        state NORMAL
          en:state_AirFilter = NORMAL;
        (default) -> FAULT_DETECTION.TIME_BASED.NORMAL.DEBOUNCE
        FAULT_DETECTION.TIME_BASED.NORMAL.DEBOUNCE -> FAULT_DETECTION.TIME_BASED.NORMAL.NORMAL : [(debounceCount >= CD_AIR_FILTER_DEBOUNCE_NORMAL)...
            | || (state_AirFilter == NORMAL)]
      state PLUGGED
        state DEBOUNCE
          en:debounceCount = 0;
          du:debounceCount++;
        state PLUGGED
          en:state_AirFilter = PLUGGED;
        (default) -> FAULT_DETECTION.TIME_BASED.PLUGGED.DEBOUNCE
        FAULT_DETECTION.TIME_BASED.PLUGGED.DEBOUNCE -> FAULT_DETECTION.TIME_BASED.PLUGGED.PLUGGED : [(debounceCount >= CD_AIR_FILTER_DEBOUNCE_PLUGGED)]
      (default) -> FAULT_DETECTION.TIME_BASED.NORMAL
      FAULT_DETECTION.TIME_BASED.NORMAL -> FAULT_DETECTION.TIME_BASED.NOT_CONNECTED [order 2] : [(din_AirFilter == ACTIVE)...
          | && ((state_Engine == ENGINE_NOT_RUNNING) || (state_AirFilter == NOT_CONNECTED))]
      FAULT_DETECTION.TIME_BASED.NOT_CONNECTED -> FAULT_DETECTION.TIME_BASED.NORMAL : [(din_AirFilter != ACTIVE)]
      FAULT_DETECTION.TIME_BASED.NORMAL -> FAULT_DETECTION.TIME_BASED.PLUGGED [order 1] : [(din_AirFilter == ACTIVE)...
          | && (state_Engine == ENGINE_RUNNING)...
          | && (state_AirFilter != NOT_CONNECTED)]
      FAULT_DETECTION.TIME_BASED.PLUGGED -> FAULT_DETECTION.TIME_BASED.NORMAL : [(din_AirFilter != ACTIVE)...
          | && (state_AirFilter == NORMAL)]
    (default) -> NON_FUNCTIONAL
    NON_FUNCTIONAL -> COUNTER_BASED [order 1] : [(CD_AIR_FILTER_FUNCTION == 1)]
    NON_FUNCTIONAL -> TIME_BASED [order 2] : [(CD_AIR_FILTER_FUNCTION != NON_FUNCTIONAL)]
  data: din_AirFilter input uint8; state_Engine input uint8; state_AirFilter output uint8; CD_AIR_FILTER_DEBOUNCE_NORMAL parameter uint16; CD_AIR_FILTER_DEBOUNCE_NOT_CONNECTED parameter uint16; CD_AIR_FILTER_DEBOUNCE_PLUGGED parameter uint16; CD_AIR_FILTER_FUNCTION parameter uint8; debounceCount local uint16 =0; ACTIVE parameter uint8; ENGINE_NOT_RUNNING parameter uint8; PLUGGED parameter uint8; NON_FUNCTIONAL parameter uint8; ENGINE_RUNNING parameter uint8; NORMAL parameter uint8; NOT_CONNECTED parameter uint8; count_AFPlugged output uint16; state_Engine_old local uint8; CD_AIR_FILTER_PLUGGED_WEIGHT parameter uint8; CD_AIR_FILTER_UNPLUGGED_WEIGHT parameter uint8

== stateflow chart MX_Gtwy_Control/Inputs/Aux_Pot/Aux_Pot/Aux_Pot ==
  state Aux_Pot_Neutral_Check [parallel]
    settings: executionOrder=1
    state Initialize
      en:
      state_AuxPotCal = NORMAL;
      offset_AuxPotCal = 0;
    state WaitForPress
    state Wait_For_New_Cal
    note: if we are already NOT_IN_NEUTRAL
        | be sure to not beep again
    note: set the triple beep here instead of in the fault code
        | to be sure other aux beeps don't override the fault code beep
    (default) -> Initialize
    Initialize -> WaitForPress
    WaitForPress -> (junction 4113) [order 1] : [(aux_Flow == AUX_FLOW_LOW || aux_Flow == AUX_FLOW_MEDIUM || aux_Flow == AUX_FLOW_STANDARD || aux_Flow == AUX_FLOW_HIGH)...
        | && state_Console == ACTIVE...
        | && state_Engine != ENGINE_CRANKING...
        | && state_AuxPot != OORL...
        | && state_AuxPot != OORH...
        | && state_SensorSupply == NORMAL]
    (junction 4113) -> (junction 4114)
    (junction 4114) -> (junction 4115) [order 1] : [rm_AuxPot >= CD_AUXPOT_CAL_DEADBAND_LOW...
        |  && rm_AuxPot <= CD_AUXPOT_CAL_DEADBAND_HIGH]
    (junction 4118) -> (junction 4113)
    (junction 4114) -> (junction 4116) [order 2]
    (junction 4115) -> (junction 4119) : {
        | state_AuxPotCal = NORMAL;
        | offset_AuxPotCal = int16(CD_AUXPOT_CENTER_RATIO) - rm_AuxPot;
        | }
    (junction 4116) -> (junction 4117) [order 1] : [state_AuxPot != NOT_IN_NEUTRAL]
    (junction 4116) -> (junction 4120) [order 2] : {
        | state_Buzzer = BEEP_NONE;
        | }
    (junction 4117) -> (junction 4121) : {
        | state_AuxPotCal = NOT_IN_NEUTRAL;
        | offset_AuxPotCal = 0;
        | state_Buzzer = TRIPLE_SHORT_BEEP;
        | }
    WaitForPress -> (junction 4118) [order 2] : [(aux_DebounceButton || aux_DetentHold)...
        | && (state_AuxEnable == INACTIVE || aux_Flow == AUX_FLOW_INACTIVE)...
        | && state_AuxPot != OORL...
        | && state_AuxPot != OORH...
        | && state_SensorSupply == NORMAL]
    (junction 4122) -> (junction 4118)
    (junction 4119) -> Wait_For_New_Cal
    (junction 4120) -> (junction 4121)
    (junction 4121) -> Wait_For_New_Cal
    Wait_For_New_Cal -> (junction 4122) [order 2] : [(aux_DebounceButton || aux_DetentHold)...
        | && (state_AuxEnable == INACTIVE || aux_Flow == AUX_FLOW_INACTIVE)...
        | && state_AuxPot != OORL...
        | && state_AuxPot != OORH...
        | && state_SensorSupply == NORMAL]
    Wait_For_New_Cal -> WaitForPress [order 1] : [state_Console != ACTIVE...
        | || state_Engine == ENGINE_CRANKING...
        | || state_AuxPot == OORL ...
        | || state_AuxPot ==OORH ...
        | || state_SensorSupply != NORMAL]
  state Aux_Pot_State [parallel]
    settings: executionOrder=2
    state Supply_OK
      state NORMAL
        en:
        position_AuxPotIn = rm_AuxPot + offset_AuxPotCal;
        position_AuxPotOut = position_AuxPotIn;
        du:
        position_AuxPotIn = rm_AuxPot + offset_AuxPotCal;
        position_AuxPotOut = position_AuxPotIn;
        (history)
        state Neutral
          en:
          state_AuxPot = NEUTRAL;
        state Rod_Active
          en:
          state_AuxPot =...
          ROD_ACTIVE;
        state Base_Active
          en:
          state_AuxPot =...
          BASE_ACTIVE;
        (default) -> Neutral
        Neutral -> Base_Active [order 2] : [position_AuxPotIn < base_Threshold]
        Neutral -> Rod_Active [order 1] : [position_AuxPotIn > rod_Threshold]
        Rod_Active -> Neutral [order 2] : [position_AuxPotIn <= rod_Threshold]
        Base_Active -> Neutral [order 2] : [position_AuxPotIn >= base_Threshold]
        Rod_Active -> Base_Active [order 1] : [position_AuxPotIn <= rod_Threshold...
            |  && position_AuxPotIn < base_Threshold]
        Base_Active -> Rod_Active [order 1] : [position_AuxPotIn >= rod_Threshold...
            |  && position_AuxPotIn > base_Threshold]
      state OORH
        en:
        state_AuxPot = OORH;
      state debounce_OORH
        en:
        position_AuxPotOut =...
        CD_AUXPOT_CENTER_RATIO;
      state OORL
        en:
        state_AuxPot = OORL;
      state debounce_OORL
        en:
        position_AuxPotOut =...
        CD_AUXPOT_CENTER_RATIO;
      state NOT_IN_NEUTRAL
        en:
        state_AuxPot = NOT_IN_NEUTRAL;
        position_AuxPotOut =...
        CD_AUXPOT_CENTER_RATIO;
      (default) -> NORMAL
      NORMAL -> debounce_OORH [order 1] : [rm_AuxPot > CD_AUXPOT_OORH]
      debounce_OORH -> OORH [order 1] : [after(CD_AUXPOT_OOR_DB,tick)]
      debounce_OORH -> NORMAL [order 2] : [rm_AuxPot <= CD_AUXPOT_OORH]
      OORH -> NORMAL : [rm_AuxPot <= CD_AUXPOT_OORH]
      NORMAL -> debounce_OORL [order 2] : [rm_AuxPot < CD_AUXPOT_OORL]
      debounce_OORL -> OORL [order 1] : [after(CD_AUXPOT_OOR_DB,tick)]
      debounce_OORL -> NORMAL [order 2] : [rm_AuxPot >= CD_AUXPOT_OORL]
      OORL -> NORMAL : [rm_AuxPot >= CD_AUXPOT_OORL]
      NORMAL -> NOT_IN_NEUTRAL [order 3] : [state_AuxPotCal == NOT_IN_NEUTRAL]
      NOT_IN_NEUTRAL -> NORMAL : [state_AuxPotCal != NOT_IN_NEUTRAL]
    state Supply_Fault
      en:
      state_AuxPot = NORMAL;
      position_AuxPotOut =...
      CD_AUXPOT_CENTER_RATIO;
    (default) -> Supply_OK : {
        | base_Threshold = CD_AUXPOT_CENTER_RATIO - CD_AUXPOT_DEADBAND;
        | rod_Threshold = CD_AUXPOT_CENTER_RATIO + CD_AUXPOT_DEADBAND;
        | }
    Supply_OK -> Supply_Fault : [state_SensorSupply != NORMAL]
    Supply_Fault -> Supply_OK : [state_SensorSupply == NORMAL]
  data: aux_DebounceButton input uint8; aux_Flow input uint8; state_AuxEnable input uint8; aux_DetentHold input uint8; state_AuxPot output uint8; position_AuxPotOut output int16; position_AuxPotIn local int16 size=1; offset_AuxPotCal local int16; state_AuxPotCal local uint8; CD_AUXPOT_CENTER_RATIO parameter uint16; CD_AUXPOT_CAL_DEADBAND_HIGH parameter uint16; CD_AUXPOT_CAL_DEADBAND_LOW parameter uint16; CD_AUXPOT_OORH parameter uint16; CD_AUXPOT_OORL parameter uint16; NOT_IN_NEUTRAL parameter uint8; OORH parameter uint8; OORL parameter uint8; CD_AUXPOT_OOR_DB parameter uint8; BASE_ACTIVE parameter uint8; base_Threshold local uint16; CD_AUXPOT_DEADBAND parameter uint8; NEUTRAL parameter uint8; ROD_ACTIVE parameter uint8; rod_Threshold local uint16; NORMAL parameter uint8; INACTIVE parameter uint8; ACTIVE parameter uint8; state_Console input uint8; state_Engine input uint8; state_SensorSupply input uint8; rm_AuxPot input int16; ENGINE_CRANKING parameter uint8; TRIPLE_SHORT_BEEP parameter uint8; state_Buzzer data_store_memory double; BEEP_NONE parameter uint8; AUX_FLOW_LOW parameter uint8; AUX_FLOW_MEDIUM parameter uint8; AUX_FLOW_STANDARD parameter uint8; AUX_FLOW_HIGH parameter uint8; AUX_FLOW_INACTIVE parameter uint8

== stateflow chart MX_Gtwy_Control/System/Keyless_Entry/Password_Entry ==
  state INIT
    en:
    user_KeylessEntry = UNDEFINED;
    state_KeylessEntryLock = KE_UNKNOWN;
    state_KeylessEntryPassword = KE_UNKNOWN;
  state CLEAR_BUFFER
    note: Set first byte in buffer to undefined so that an empty
        | password will not pass, but leave remaining null so
        | that unused digits will pass as null.
    (default) -> (junction 4227)
    (junction 4228) -> (junction 4226) [order 1] : [position < KE_PASSWORD_SIZE]
    (junction 4226) -> (junction 4229) : {buffer_password[position] = 0;}
    (junction 4227) -> (junction 4228) : {position = 0;}
    (junction 4229) -> (junction 4228) : {position++;}
    (junction 4228) -> (junction 4230) [order 2] : {
        | position = 0;
        | buffer_password[0] = UNDEFINED;
        | }
  state WAIT_FOR_KEYPRESS
    en:
    state_Buzzer = BEEP_NONE;
  state CAPTURE_KEYPRESS
    state BUTTON_9
      en:buffer_password[position] = ASCII_9;
      en:position++;
    state BUTTON_8
      en:buffer_password[position] = ASCII_8;
      en:position++;
    state BUTTON_7
      en:buffer_password[position] = ASCII_7;
      en:position++;
    state BUTTON_0
      en:buffer_password[position] = ASCII_0;
      en:position++;
    state NONE
      en:
      state_KeylessEntryPassword = KE_UNKNOWN;
      ex:
      state_Buzzer = SINGLE_SHORT_BEEP;
    state BUTTON_1
      en:buffer_password[position] = ASCII_1;
      en:position++;
    state BUTTON_6
      en:buffer_password[position] = ASCII_6;
      en:position++;
    state BUTTON_5
      en:buffer_password[position] = ASCII_5;
      en:position++;
    state BUTTON_2
      en:buffer_password[position] = ASCII_2;
      en:position++;
    state BUTTON_4
      en:buffer_password[position] = ASCII_4;
      en:position++;
    state BUTTON_3
      en:buffer_password[position] = ASCII_3;
      en:position++;
    NONE -> BUTTON_9 [order 1] : [((can_KeylessEntry[2] & KEYPAD_MASK_9) == KEYPAD_PRESSED_9)]
    NONE -> BUTTON_8 [order 2] : [((can_KeylessEntry[1] & KEYPAD_MASK_8) == KEYPAD_PRESSED_8)]
    NONE -> BUTTON_7 [order 3] : [((can_KeylessEntry[1] & KEYPAD_MASK_7) == KEYPAD_PRESSED_7)]
    NONE -> BUTTON_0 [order 10] : [((can_KeylessEntry[2] & KEYPAD_MASK_0) == KEYPAD_PRESSED_0)]
    (default) -> NONE
    NONE -> BUTTON_6 [order 4] : [((can_KeylessEntry[1] & KEYPAD_MASK_6) == KEYPAD_PRESSED_6)]
    NONE -> BUTTON_1 [order 9] : [((can_KeylessEntry[0] & KEYPAD_MASK_1) == KEYPAD_PRESSED_1)]
    NONE -> BUTTON_2 [order 8] : [((can_KeylessEntry[0] & KEYPAD_MASK_2) == KEYPAD_PRESSED_2)]
    NONE -> BUTTON_5 [order 5] : [((can_KeylessEntry[1] & KEYPAD_MASK_5) == KEYPAD_PRESSED_5)]
    NONE -> BUTTON_3 [order 7] : [((can_KeylessEntry[0] & KEYPAD_MASK_3) == KEYPAD_PRESSED_3)]
    NONE -> BUTTON_4 [order 6] : [((can_KeylessEntry[0] & KEYPAD_MASK_4) == KEYPAD_PRESSED_4)]
  state CHECK_PASSWORD
    (default) -> (junction 4248)
    (junction 4248) -> (junction 4250) : {
        | user = 0;
        | position = 0;
        |
        | }
    (junction 4252) -> (junction 4249) [order 1] : %all positions match
        | [position == KE_PASSWORD_SIZE - 1]
    (junction 4250) -> (junction 4251) [order 1] : %check each user
        | [user < NUMBER_OF_KE_PASSWORDS]
    (junction 4251) -> (junction 4252) [order 1] : %check each position for match
        | [buffer_password[position] == ee_KeylessEntryPasswords[position][user]]
    (junction 4254) -> (junction 4251) : {
        | position++;
        | }
    (junction 4253) -> (junction 4250) : {
        | user++;
        | position = 0;
        | }
    (junction 4249) -> (junction 4256) : %authenticated
        | {
        | state_KeylessEntryPassword = KE_PASS;
        | user_KeylessEntry = user;
        | }
    (junction 4251) -> (junction 4253) [order 2]
    (junction 4252) -> (junction 4254) [order 2]
    (junction 4250) -> (junction 4255) [order 2] : %no matches
        | {
        | state_KeylessEntryPassword = KE_FAIL;
        | }
    (junction 4256) -> (junction 4255)
    (junction 4255) -> (junction 4257)
  state AUTHENTICATED
    state LOCK
      en:
      state_KeylessEntryLock = KE_LOCK;
    state UNLOCK
      entry: state_KeylessEntryLock = KE_UNLOCK;
    note: force delay between switching of locked and unlocked to keep in sync
        | with secondary controller... cannot switch locked state until after door has actually
        | locked or unlocked
    (default) -> (junction 4271)
    (junction 4271) -> (junction 4272) [order 2]
    (junction 4271) -> LOCK [order 1] : [lock]
    (junction 4272) -> UNLOCK
    LOCK -> UNLOCK : [(can_KeylessEntry[2] & KEYPAD_UNLOCK_MASK) == KEYPAD_UNLOCK_PRESSED...
        | && after(CD_DOOR_LOCK_PULSE_LENGTH, tick)]
    UNLOCK -> LOCK : [(can_KeylessEntry[2] & KEYPAD_LOCK_MASK) == KEYPAD_LOCK_PRESSED...
        | && after(CD_DOOR_LOCK_PULSE_LENGTH, tick)]
  (default) -> INIT
  INIT -> CLEAR_BUFFER
  CHECK_PASSWORD -> CLEAR_BUFFER [order 1] : [state_KeylessEntryPassword == KE_FAIL]
      | {
      | state_Buzzer = TRIPLE_SHORT_BEEP;
      | }
  CLEAR_BUFFER -> WAIT_FOR_KEYPRESS
  WAIT_FOR_KEYPRESS -> CAPTURE_KEYPRESS [order 1] : [((can_KeylessEntry[0] != 0) || (can_KeylessEntry[1] != 0) || ((can_KeylessEntry[2] & 0x0F) != 0))...
      | && position < KE_PASSWORD_SIZE]
  CAPTURE_KEYPRESS -> WAIT_FOR_KEYPRESS : [(can_KeylessEntry[0] == 0)...
      | && (can_KeylessEntry[1] == 0)...
      | && (can_KeylessEntry[2] == 0)]
  WAIT_FOR_KEYPRESS -> CHECK_PASSWORD [order 2] : [(can_KeylessEntry[2] & KEYPAD_LOCK_MASK) == KEYPAD_LOCK_PRESSED]{lock = 1;}
  WAIT_FOR_KEYPRESS -> CHECK_PASSWORD [order 3] : [(can_KeylessEntry[2] & KEYPAD_UNLOCK_MASK) == KEYPAD_UNLOCK_PRESSED]{lock = 0;}
  CHECK_PASSWORD -> AUTHENTICATED [order 2] : [state_KeylessEntryPassword == KE_PASS]
  data: state_KeylessEntryLock output uint8; KEYPAD_LOCK_MASK parameter uint8; KEYPAD_LOCK_PRESSED parameter uint8; KEYPAD_UNLOCK_MASK parameter uint8; KEYPAD_UNLOCK_PRESSED parameter uint8; KE_PASS parameter uint8; KE_FAIL parameter uint8; KE_LOCK parameter uint8; KE_UNLOCK parameter uint8; user_KeylessEntry output uint8; ASCII_1 parameter uint8; ASCII_2 parameter uint8; ASCII_3 parameter uint8; ASCII_4 parameter uint8; ASCII_5 parameter uint8; ASCII_6 parameter uint8; ASCII_7 parameter uint8; ASCII_8 parameter uint8; ASCII_9 parameter uint8; ASCII_0 parameter uint8; KEYPAD_PRESSED_1 parameter uint8; KEYPAD_MASK_1 parameter uint8; KEYPAD_MASK_2 parameter uint8; KEYPAD_PRESSED_2 parameter uint8; KEYPAD_MASK_3 parameter uint8; KEYPAD_PRESSED_3 parameter uint8; KEYPAD_MASK_4 parameter uint8; KEYPAD_PRESSED_4 parameter uint8; KEYPAD_MASK_5 parameter uint8; KEYPAD_PRESSED_5 parameter uint8; KEYPAD_MASK_6 parameter uint8; KEYPAD_PRESSED_6 parameter uint8; KEYPAD_MASK_7 parameter uint8; KEYPAD_PRESSED_7 parameter uint8; KEYPAD_MASK_8 parameter uint8; KEYPAD_PRESSED_8 parameter uint8; KEYPAD_MASK_9 parameter uint8; KEYPAD_PRESSED_9 parameter uint8; KEYPAD_MASK_0 parameter uint8; KEYPAD_PRESSED_0 parameter uint8; KE_PASSWORD_SIZE parameter uint8; ee_KeylessEntryPasswords input uint8 size=[10,22]; can_KeylessEntry input uint8 size=3; buffer_password local uint8 size=10; lock local boolean; KE_UNKNOWN parameter uint8; NUMBER_OF_KE_PASSWORDS parameter uint8; state_KeylessEntryPassword output uint8; state_Buzzer data_store_memory uint8; position local uint8; user local uint8; BEEP_NONE parameter uint8; SINGLE_SHORT_BEEP parameter uint8; TRIPLE_SHORT_BEEP parameter uint8; UNDEFINED parameter uint8; CD_DOOR_LOCK_PULSE_LENGTH parameter uint16

== stateflow chart MX_Gtwy_Control/Inputs/Load_Moment/Load_Moment_Switch/Load_Moment ==
  state Normal
    en:
    state_LoadMoment = NORMAL;
  state Normal_DB
  state Error_DB
  state InError
    en:
    state_LoadMoment = IN_ERROR;
    du:
    state_Buzzer = CONTINUOUS_BEEP;
  (default) -> Normal
  Normal -> Error_DB : [ain_LoadMoment > CD_LOAD_MOMENT_ON]
  Error_DB -> Normal [order 1] : [ain_LoadMoment < CD_LOAD_MOMENT_OFF]
  Normal_DB -> Normal [order 1] : [after(CD_LOAD_MOMENT_NORMAL_DB,tick)]
      | {state_Buzzer = NO_BEEP;}
  InError -> Normal_DB : [ain_LoadMoment < CD_LOAD_MOMENT_OFF]
  Normal_DB -> InError [order 2] : [ain_LoadMoment > CD_LOAD_MOMENT_ON]
  Error_DB -> InError [order 2] : [after(CD_LOAD_MOMENT_ERROR_DB,tick)]
  data: NORMAL parameter uint8; CD_LOAD_MOMENT_ERROR_DB parameter uint8; CD_LOAD_MOMENT_NORMAL_DB parameter uint8; IN_ERROR parameter uint8; ain_LoadMoment input uint16; state_LoadMoment output uint8; CD_LOAD_MOMENT_ON parameter uint16; CD_LOAD_MOMENT_OFF parameter uint16; state_Buzzer data_store_memory double; NO_BEEP parameter uint8; CONTINUOUS_BEEP parameter uint8
